simulink model "os_polyphase_synth_4096c"  (R2021a) (continued)
== system os_polyphase_synth_4096c/pfb_fir/pol1_in1_tap53 == (continued)
      | BCIN*ACIN+PCIN>>17+C
      | BCIN*ACIN+PCIN>>17+C+CARRYIN
      | BCIN*ACIN+PCIN>>17+CARRYIN
      | BCIN*ACIN+PCIN>>17+P
      | BCIN*ACIN+PCIN>>17+P+CARRYIN
      | BCIN*ACIN-C
      | BCIN*ACIN-P
      | BCIN*ACIN-P>>17
      | BCIN*ACIN-PCIN
      | BCIN*ACIN-PCIN>>17
      | BCIN*D
      | BCIN*D+C
      | BCIN*D+C+C
      | BCIN*D+C+C+CARRYIN
      | BCIN*D+C+CARRYIN
      | BCIN*D+C+P
      | BCIN*D+C+P+CARRYIN
      | BCIN*D+C+P>>17
      | BCIN*D+C+P>>17+CARRYIN
      | BCIN*D+C+PCIN
      | BCIN*D+C+PCIN+CARRYIN
      | BCIN*D+C+PCIN>>17
      | BCIN*D+C+PCIN>>17+CARRYIN
      | BCIN*D+C-C
      | BCIN*D+C-P
      | BCIN*D+C-P>>17
      | BCIN*D+C-PCIN
      | BCIN*D+C-PCIN>>17
      | BCIN*D+CARRYIN
      | BCIN*D+P
      | BCIN*D+P+C
      | BCIN*D+P+C+CARRYIN
      | BCIN*D+P+CARRYIN
      | BCIN*D+P+P
      | BCIN*D+P+P+CARRYIN
      | BCIN*D+P+P>>17
      | BCIN*D+P+P>>17+CARRYIN
      | BCIN*D+P+PCIN
      | BCIN*D+P+PCIN+CARRYIN
      | BCIN*D+P+PCIN>>17
      | BCIN*D+P+PCIN>>17+CARRYIN
      | BCIN*D+P-C
      | BCIN*D+P-P
      | BCIN*D+P-P>>17
      | BCIN*D+P-PCIN
      | BCIN*D+P-PCIN>>17
      | BCIN*D+P>>17
      | BCIN*D+P>>17+C
      | BCIN*D+P>>17+C+CARRYIN
      | BCIN*D+P>>17+CARRYIN
      | BCIN*D+P>>17+P
      | BCIN*D+P>>17+P+CARRYIN
      | BCIN*D+PCIN
      | BCIN*D+PCIN+C
      | BCIN*D+PCIN+C+CARRYIN
      | BCIN*D+PCIN+CARRYIN
      | BCIN*D+PCIN+P
      | BCIN*D+PCIN+P+CARRYIN
      | BCIN*D+PCIN>>17
      | BCIN*D+PCIN>>17+C
      | BCIN*D+PCIN>>17+C+CARRYIN
      | BCIN*D+PCIN>>17+CARRYIN
      | BCIN*D+PCIN>>17+P
      | BCIN*D+PCIN>>17+P+CARRYIN
      | BCIN*D-C
      | BCIN*D-P
      | BCIN*D-P>>17
      | BCIN*D-PCIN
      | BCIN*D-PCIN>>17
      | C
      | C+(A+D)
      | C+(A+D)*(A+D)
      | C+(A+D)*(A+D)+C
      | C+(A+D)*(A+D)+C+CARRYIN
      | C+(A+D)*(A+D)+CARRYIN
      | C+(A+D)*(A+D)+P
      | C+(A+D)*(A+D)+P+CARRYIN
      | C+(A+D)*(A+D)+P>>17
      | C+(A+D)*(A+D)+P>>17+CARRYIN
      | C+(A+D)*(A+D)+PCIN
      | C+(A+D)*(A+D)+PCIN+CARRYIN
      | C+(A+D)*(A+D)+PCIN>>17
      | C+(A+D)*(A+D)+PCIN>>17+CARRYIN
      | C+(A+D)*(A+D)-C
      | C+(A+D)*(A+D)-P
      | C+(A+D)*(A+D)-P>>17
      | C+(A+D)*(A+D)-PCIN
      | C+(A+D)*(A+D)-PCIN>>17
      | C+(A+D)*B
      | C+(A+D)*B+C
      | C+(A+D)*B+C+CARRYIN
      | C+(A+D)*B+CARRYIN
      | C+(A+D)*B+P
      | C+(A+D)*B+P+CARRYIN
      | C+(A+D)*B+P>>17
      | C+(A+D)*B+P>>17+CARRYIN
      | C+(A+D)*B+PCIN
      | C+(A+D)*B+PCIN+CARRYIN
      | C+(A+D)*B+PCIN>>17
      | C+(A+D)*B+PCIN>>17+CARRYIN
      | C+(A+D)*B-C
      | C+(A+D)*B-P
      | C+(A+D)*B-P>>17
      | C+(A+D)*B-PCIN
      | C+(A+D)*B-PCIN>>17
      | C+(A+D)*BCIN
      | C+(A+D)*BCIN+C
      | C+(A+D)*BCIN+C+CARRYIN
      | C+(A+D)*BCIN+CARRYIN
      | C+(A+D)*BCIN+P
      | C+(A+D)*BCIN+P+CARRYIN
      | C+(A+D)*BCIN+P>>17
      | C+(A+D)*BCIN+P>>17+CARRYIN
      | C+(A+D)*BCIN+PCIN
      | C+(A+D)*BCIN+PCIN+CARRYIN
      | C+(A+D)*BCIN+PCIN>>17
      | C+(A+D)*BCIN+PCIN>>17+CARRYIN
      | C+(A+D)*BCIN-C
      | C+(A+D)*BCIN-P
      | C+(A+D)*BCIN-P>>17
      | C+(A+D)*BCIN-PCIN
      | C+(A+D)*BCIN-PCIN>>17
      | C+(A+D)+C
      | C+(A+D)+C+CARRYIN
      | C+(A+D)+CARRYIN
      | C+(A+D)+P
      | C+(A+D)+P+CARRYIN
      | C+(A+D)+P>>17
      | C+(A+D)+P>>17+CARRYIN
      | C+(A+D)+PCIN
      | C+(A+D)+PCIN+CARRYIN
      | C+(A+D)+PCIN>>17
      | C+(A+D)+PCIN>>17+CARRYIN
      | C+(A+D)-C
      | C+(A+D)-P
      | C+(A+D)-P>>17
      | C+(A+D)-PCIN
      | C+(A+D)-PCIN>>17
      | C+(ACIN+D)
      | C+(ACIN+D)*(ACIN+D)
      | C+(ACIN+D)*(ACIN+D)+C
      | C+(ACIN+D)*(ACIN+D)+C+CARRYIN
      | C+(ACIN+D)*(ACIN+D)+CARRYIN
      | C+(ACIN+D)*(ACIN+D)+P
      | C+(ACIN+D)*(ACIN+D)+P+CARRYIN
      | C+(ACIN+D)*(ACIN+D)+P>>17
      | C+(ACIN+D)*(ACIN+D)+P>>17+CARRYIN
      | C+(ACIN+D)*(ACIN+D)+PCIN
      | C+(ACIN+D)*(ACIN+D)+PCIN+CARRYIN
      | C+(ACIN+D)*(ACIN+D)+PCIN>>17
      | C+(ACIN+D)*(ACIN+D)+PCIN>>17+CARRYIN
      | C+(ACIN+D)*(ACIN+D)-C
      | C+(ACIN+D)*(ACIN+D)-P
      | C+(ACIN+D)*(ACIN+D)-P>>17
      | C+(ACIN+D)*(ACIN+D)-PCIN
      | C+(ACIN+D)*(ACIN+D)-PCIN>>17
      | C+(ACIN+D)*B
      | C+(ACIN+D)*B+C
      | C+(ACIN+D)*B+C+CARRYIN
      | C+(ACIN+D)*B+CARRYIN
      | C+(ACIN+D)*B+P
      | C+(ACIN+D)*B+P+CARRYIN
      | C+(ACIN+D)*B+P>>17
      | C+(ACIN+D)*B+P>>17+CARRYIN
      | C+(ACIN+D)*B+PCIN
      | C+(ACIN+D)*B+PCIN+CARRYIN
      | C+(ACIN+D)*B+PCIN>>17
      | C+(ACIN+D)*B+PCIN>>17+CARRYIN
      | C+(ACIN+D)*B-C
      | C+(ACIN+D)*B-P
      | C+(ACIN+D)*B-P>>17
      | C+(ACIN+D)*B-PCIN
      | C+(ACIN+D)*B-PCIN>>17
      | C+(ACIN+D)*BCIN
      | C+(ACIN+D)*BCIN+C
      | C+(ACIN+D)*BCIN+C+CARRYIN
      | C+(ACIN+D)*BCIN+CARRYIN
      | C+(ACIN+D)*BCIN+P
      | C+(ACIN+D)*BCIN+P+CARRYIN
      | C+(ACIN+D)*BCIN+P>>17
      | C+(ACIN+D)*BCIN+P>>17+CARRYIN
      | C+(ACIN+D)*BCIN+PCIN
      | C+(ACIN+D)*BCIN+PCIN+CARRYIN
      | C+(ACIN+D)*BCIN+PCIN>>17
      | C+(ACIN+D)*BCIN+PCIN>>17+CARRYIN
      | C+(ACIN+D)*BCIN-C
      | C+(ACIN+D)*BCIN-P
      | C+(ACIN+D)*BCIN-P>>17
      | C+(ACIN+D)*BCIN-PCIN
      | C+(ACIN+D)*BCIN-PCIN>>17
      | C+(ACIN+D)+C
      | C+(ACIN+D)+C+CARRYIN
      | C+(ACIN+D)+CARRYIN
      | C+(ACIN+D)+P
      | C+(ACIN+D)+P+CARRYIN
      | C+(ACIN+D)+P>>17
      | C+(ACIN+D)+P>>17+CARRYIN
      | C+(ACIN+D)+PCIN
      | C+(ACIN+D)+PCIN+CARRYIN
      | C+(ACIN+D)+PCIN>>17
      | C+(ACIN+D)+PCIN>>17+CARRYIN
      | C+(ACIN+D)-C
      | C+(ACIN+D)-P
      | C+(ACIN+D)-P>>17
      | C+(ACIN+D)-PCIN
      | C+(ACIN+D)-PCIN>>17
      | C+(D+A)
      | C+(D+A)*(D+A)
      | C+(D+A)*(D+A)+C
      | C+(D+A)*(D+A)+C+CARRYIN
      | C+(D+A)*(D+A)+CARRYIN
      | C+(D+A)*(D+A)+P
      | C+(D+A)*(D+A)+P+CARRYIN
      | C+(D+A)*(D+A)+P>>17
      | C+(D+A)*(D+A)+P>>17+CARRYIN
      | C+(D+A)*(D+A)+PCIN
      | C+(D+A)*(D+A)+PCIN+CARRYIN
      | C+(D+A)*(D+A)+PCIN>>17
      | C+(D+A)*(D+A)+PCIN>>17+CARRYIN
      | C+(D+A)*(D+A)-C
      | C+(D+A)*(D+A)-P
      | C+(D+A)*(D+A)-P>>17
      | C+(D+A)*(D+A)-PCIN
      | C+(D+A)*(D+A)-PCIN>>17
      | C+(D+A)*B
      | C+(D+A)*B+C
      | C+(D+A)*B+C+CARRYIN
      | C+(D+A)*B+CARRYIN
      | C+(D+A)*B+P
      | C+(D+A)*B+P+CARRYIN
      | C+(D+A)*B+P>>17
      | C+(D+A)*B+P>>17+CARRYIN
      | C+(D+A)*B+PCIN
      | C+(D+A)*B+PCIN+CARRYIN
      | C+(D+A)*B+PCIN>>17
      | C+(D+A)*B+PCIN>>17+CARRYIN
      | C+(D+A)*B-C
      | C+(D+A)*B-P
      | C+(D+A)*B-P>>17
      | C+(D+A)*B-PCIN
      | C+(D+A)*B-PCIN>>17
      | C+(D+A)*BCIN
      | C+(D+A)*BCIN+C
      | C+(D+A)*BCIN+C+CARRYIN
      | C+(D+A)*BCIN+CARRYIN
      | C+(D+A)*BCIN+P
      | C+(D+A)*BCIN+P+CARRYIN
      | C+(D+A)*BCIN+P>>17
      | C+(D+A)*BCIN+P>>17+CARRYIN
      | C+(D+A)*BCIN+PCIN
      | C+(D+A)*BCIN+PCIN+CARRYIN
      | C+(D+A)*BCIN+PCIN>>17
      | C+(D+A)*BCIN+PCIN>>17+CARRYIN
      | C+(D+A)*BCIN-C
      | C+(D+A)*BCIN-P
      | C+(D+A)*BCIN-P>>17
      | C+(D+A)*BCIN-PCIN
      | C+(D+A)*BCIN-PCIN>>17
      | C+(D+A)+C
      | C+(D+A)+C+CARRYIN
      | C+(D+A)+CARRYIN
      | C+(D+A)+P
      | C+(D+A)+P+CARRYIN
      | C+(D+A)+P>>17
      | C+(D+A)+P>>17+CARRYIN
      | C+(D+A)+PCIN
      | C+(D+A)+PCIN+CARRYIN
      | C+(D+A)+PCIN>>17
      | C+(D+A)+PCIN>>17+CARRYIN
      | C+(D+A)-C
      | C+(D+A)-P
      | C+(D+A)-P>>17
      | C+(D+A)-PCIN
      | C+(D+A)-PCIN>>17
      | C+(D+ACIN)
      | C+(D+ACIN)*(D+ACIN)
      | C+(D+ACIN)*(D+ACIN)+C
      | C+(D+ACIN)*(D+ACIN)+C+CARRYIN
      | C+(D+ACIN)*(D+ACIN)+CARRYIN
      | C+(D+ACIN)*(D+ACIN)+P
      | C+(D+ACIN)*(D+ACIN)+P+CARRYIN
      | C+(D+ACIN)*(D+ACIN)+P>>17
      | C+(D+ACIN)*(D+ACIN)+P>>17+CARRYIN
      | C+(D+ACIN)*(D+ACIN)+PCIN
      | C+(D+ACIN)*(D+ACIN)+PCIN+CARRYIN
      | C+(D+ACIN)*(D+ACIN)+PCIN>>17
      | C+(D+ACIN)*(D+ACIN)+PCIN>>17+CARRYIN
      | C+(D+ACIN)*(D+ACIN)-C
      | C+(D+ACIN)*(D+ACIN)-P
      | C+(D+ACIN)*(D+ACIN)-P>>17
      | C+(D+ACIN)*(D+ACIN)-PCIN
      | C+(D+ACIN)*(D+ACIN)-PCIN>>17
      | C+(D+ACIN)*B
      | C+(D+ACIN)*B+C
      | C+(D+ACIN)*B+C+CARRYIN
      | C+(D+ACIN)*B+CARRYIN
      | C+(D+ACIN)*B+P
      | C+(D+ACIN)*B+P+CARRYIN
      | C+(D+ACIN)*B+P>>17
      | C+(D+ACIN)*B+P>>17+CARRYIN
      | C+(D+ACIN)*B+PCIN
      | C+(D+ACIN)*B+PCIN+CARRYIN
      | C+(D+ACIN)*B+PCIN>>17
      | C+(D+ACIN)*B+PCIN>>17+CARRYIN
      | C+(D+ACIN)*B-C
      | C+(D+ACIN)*B-P
      | C+(D+ACIN)*B-P>>17
      | C+(D+ACIN)*B-PCIN
      | C+(D+ACIN)*B-PCIN>>17
      | C+(D+ACIN)*BCIN
      | C+(D+ACIN)*BCIN+C
      | C+(D+ACIN)*BCIN+C+CARRYIN
      | C+(D+ACIN)*BCIN+CARRYIN
      | C+(D+ACIN)*BCIN+P
      | C+(D+ACIN)*BCIN+P+CARRYIN
      | C+(D+ACIN)*BCIN+P>>17
      | C+(D+ACIN)*BCIN+P>>17+CARRYIN
      | C+(D+ACIN)*BCIN+PCIN
      | C+(D+ACIN)*BCIN+PCIN+CARRYIN
      | C+(D+ACIN)*BCIN+PCIN>>17
      | C+(D+ACIN)*BCIN+PCIN>>17+CARRYIN
      | C+(D+ACIN)*BCIN-C
      | C+(D+ACIN)*BCIN-P
      | C+(D+ACIN)*BCIN-P>>17
      | C+(D+ACIN)*BCIN-PCIN
      | C+(D+ACIN)*BCIN-PCIN>>17
      | C+(D+ACIN)+C
      | C+(D+ACIN)+C+CARRYIN
      | C+(D+ACIN)+CARRYIN
      | C+(D+ACIN)+P
      | C+(D+ACIN)+P+CARRYIN
      | C+(D+ACIN)+P>>17
      | C+(D+ACIN)+P>>17+CARRYIN
      | C+(D+ACIN)+PCIN
      | C+(D+ACIN)+PCIN+CARRYIN
      | C+(D+ACIN)+PCIN>>17
      | C+(D+ACIN)+PCIN>>17+CARRYIN
      | C+(D+ACIN)-C
      | C+(D+ACIN)-P
      | C+(D+ACIN)-P>>17
      | C+(D+ACIN)-PCIN
      | C+(D+ACIN)-PCIN>>17
      | C+(D-A)
      | C+(D-A)*(D-A)
      | C+(D-A)*(D-A)+C
      | C+(D-A)*(D-A)+C+CARRYIN
      | C+(D-A)*(D-A)+CARRYIN
      | C+(D-A)*(D-A)+P
      | C+(D-A)*(D-A)+P+CARRYIN
      | C+(D-A)*(D-A)+P>>17
      | C+(D-A)*(D-A)+P>>17+CARRYIN
      | C+(D-A)*(D-A)+PCIN
      | C+(D-A)*(D-A)+PCIN+CARRYIN
      | C+(D-A)*(D-A)+PCIN>>17
      | C+(D-A)*(D-A)+PCIN>>17+CARRYIN
      | C+(D-A)*(D-A)-C
      | C+(D-A)*(D-A)-P
      | C+(D-A)*(D-A)-P>>17
      | C+(D-A)*(D-A)-PCIN
      | C+(D-A)*(D-A)-PCIN>>17
      | C+(D-A)*B
      | C+(D-A)*B+C
      | C+(D-A)*B+C+CARRYIN
      | C+(D-A)*B+CARRYIN
      | C+(D-A)*B+P
      | C+(D-A)*B+P+CARRYIN
      | C+(D-A)*B+P>>17
      | C+(D-A)*B+P>>17+CARRYIN
      | C+(D-A)*B+PCIN
      | C+(D-A)*B+PCIN+CARRYIN
      | C+(D-A)*B+PCIN>>17
      | C+(D-A)*B+PCIN>>17+CARRYIN
      | C+(D-A)*B-C
      | C+(D-A)*B-P
      | C+(D-A)*B-P>>17
      | C+(D-A)*B-PCIN
      | C+(D-A)*B-PCIN>>17
      | C+(D-A)*BCIN
      | C+(D-A)*BCIN+C
      | C+(D-A)*BCIN+C+CARRYIN
      | C+(D-A)*BCIN+CARRYIN
      | C+(D-A)*BCIN+P
      | C+(D-A)*BCIN+P+CARRYIN
      | C+(D-A)*BCIN+P>>17
      | C+(D-A)*BCIN+P>>17+CARRYIN
      | C+(D-A)*BCIN+PCIN
      | C+(D-A)*BCIN+PCIN+CARRYIN
      | C+(D-A)*BCIN+PCIN>>17
      | C+(D-A)*BCIN+PCIN>>17+CARRYIN
      | C+(D-A)*BCIN-C
      | C+(D-A)*BCIN-P
      | C+(D-A)*BCIN-P>>17
      | C+(D-A)*BCIN-PCIN
      | C+(D-A)*BCIN-PCIN>>17
      | C+(D-A)+C
      | C+(D-A)+C+CARRYIN
      | C+(D-A)+CARRYIN
      | C+(D-A)+P
      | C+(D-A)+P+CARRYIN
      | C+(D-A)+P>>17
      | C+(D-A)+P>>17+CARRYIN
      | C+(D-A)+PCIN
      | C+(D-A)+PCIN+CARRYIN
      | C+(D-A)+PCIN>>17
      | C+(D-A)+PCIN>>17+CARRYIN
      | C+(D-A)-C
      | C+(D-A)-P
      | C+(D-A)-P>>17
      | C+(D-A)-PCIN
      | C+(D-A)-PCIN>>17
      | C+(D-ACIN)
      | C+(D-ACIN)*(D-ACIN)
      | C+(D-ACIN)*(D-ACIN)+C
      | C+(D-ACIN)*(D-ACIN)+C+CARRYIN
      | C+(D-ACIN)*(D-ACIN)+CARRYIN
      | C+(D-ACIN)*(D-ACIN)+P
      | C+(D-ACIN)*(D-ACIN)+P+CARRYIN
      | C+(D-ACIN)*(D-ACIN)+P>>17
      | C+(D-ACIN)*(D-ACIN)+P>>17+CARRYIN
      | C+(D-ACIN)*(D-ACIN)+PCIN
      | C+(D-ACIN)*(D-ACIN)+PCIN+CARRYIN
      | C+(D-ACIN)*(D-ACIN)+PCIN>>17
      | C+(D-ACIN)*(D-ACIN)+PCIN>>17+CARRYIN
      | C+(D-ACIN)*(D-ACIN)-C
      | C+(D-ACIN)*(D-ACIN)-P
      | C+(D-ACIN)*(D-ACIN)-P>>17
      | C+(D-ACIN)*(D-ACIN)-PCIN
      | C+(D-ACIN)*(D-ACIN)-PCIN>>17
      | C+(D-ACIN)*B
      | C+(D-ACIN)*B+C
      | C+(D-ACIN)*B+C+CARRYIN
      | C+(D-ACIN)*B+CARRYIN
      | C+(D-ACIN)*B+P
      | C+(D-ACIN)*B+P+CARRYIN
      | C+(D-ACIN)*B+P>>17
      | C+(D-ACIN)*B+P>>17+CARRYIN
      | C+(D-ACIN)*B+PCIN
      | C+(D-ACIN)*B+PCIN+CARRYIN
      | C+(D-ACIN)*B+PCIN>>17
      | C+(D-ACIN)*B+PCIN>>17+CARRYIN
      | C+(D-ACIN)*B-C
      | C+(D-ACIN)*B-P
      | C+(D-ACIN)*B-P>>17
      | C+(D-ACIN)*B-PCIN
      | C+(D-ACIN)*B-PCIN>>17
      | C+(D-ACIN)*BCIN
      | C+(D-ACIN)*BCIN+C
      | C+(D-ACIN)*BCIN+C+CARRYIN
      | C+(D-ACIN)*BCIN+CARRYIN
      | C+(D-ACIN)*BCIN+P
      | C+(D-ACIN)*BCIN+P+CARRYIN
      | C+(D-ACIN)*BCIN+P>>17
      | C+(D-ACIN)*BCIN+P>>17+CARRYIN
      | C+(D-ACIN)*BCIN+PCIN
      | C+(D-ACIN)*BCIN+PCIN+CARRYIN
      | C+(D-ACIN)*BCIN+PCIN>>17
      | C+(D-ACIN)*BCIN+PCIN>>17+CARRYIN
      | C+(D-ACIN)*BCIN-C
      | C+(D-ACIN)*BCIN-P
      | C+(D-ACIN)*BCIN-P>>17
      | C+(D-ACIN)*BCIN-PCIN
      | C+(D-ACIN)*BCIN-PCIN>>17
      | C+(D-ACIN)+C
      | C+(D-ACIN)+C+CARRYIN
      | C+(D-ACIN)+CARRYIN
      | C+(D-ACIN)+P
      | C+(D-ACIN)+P+CARRYIN
      | C+(D-ACIN)+P>>17
      | C+(D-ACIN)+P>>17+CARRYIN
      | C+(D-ACIN)+PCIN
      | C+(D-ACIN)+PCIN+CARRYIN
      | C+(D-ACIN)+PCIN>>17
      | C+(D-ACIN)+PCIN>>17+CARRYIN
      | C+(D-ACIN)-C
      | C+(D-ACIN)-P
      | C+(D-ACIN)-P>>17
      | C+(D-ACIN)-PCIN
      | C+(D-ACIN)-PCIN>>17
      | C+A
      | C+A*A
      | C+A*A+C
      | C+A*A+C+CARRYIN
      | C+A*A+CARRYIN
      | C+A*A+P
      | C+A*A+P+CARRYIN
      | C+A*A+P>>17
      | C+A*A+P>>17+CARRYIN
      | C+A*A+PCIN
      | C+A*A+PCIN+CARRYIN
      | C+A*A+PCIN>>17
      | C+A*A+PCIN>>17+CARRYIN
      | C+A*A-C
      | C+A*A-P
      | C+A*A-P>>17
      | C+A*A-PCIN
      | C+A*A-PCIN>>17
      | C+A*B
      | C+A*B+C
      | C+A*B+C+CARRYIN
      | C+A*B+CARRYIN
      | C+A*B+P
      | C+A*B+P+CARRYIN
      | C+A*B+P>>17
      | C+A*B+P>>17+CARRYIN
      | C+A*B+PCIN
      | C+A*B+PCIN+CARRYIN
      | C+A*B+PCIN>>17
      | C+A*B+PCIN>>17+CARRYIN
      | C+A*B-C
      | C+A*B-P
      | C+A*B-P>>17
      | C+A*B-PCIN
      | C+A*B-PCIN>>17
      | C+A*BCIN
      | C+A*BCIN+C
      | C+A*BCIN+C+CARRYIN
      | C+A*BCIN+CARRYIN
      | C+A*BCIN+P
      | C+A*BCIN+P+CARRYIN
      | C+A*BCIN+P>>17
      | C+A*BCIN+P>>17+CARRYIN
      | C+A*BCIN+PCIN
      | C+A*BCIN+PCIN+CARRYIN
      | C+A*BCIN+PCIN>>17
      | C+A*BCIN+PCIN>>17+CARRYIN
      | C+A*BCIN-C
      | C+A*BCIN-P
      | C+A*BCIN-P>>17
      | C+A*BCIN-PCIN
      | C+A*BCIN-PCIN>>17
      | C+A+C
      | C+A+C+CARRYIN
      | C+A+CARRYIN
      | C+A+P
      | C+A+P+CARRYIN
      | C+A+P>>17
      | C+A+P>>17+CARRYIN
      | C+A+PCIN
      | C+A+PCIN+CARRYIN
      | C+A+PCIN>>17
      | C+A+PCIN>>17+CARRYIN
      | C+A-C
      | C+A-P
      | C+A-P>>17
      | C+A-PCIN
      | C+A-PCIN>>17
      | C+ACIN
      | C+ACIN*ACIN
      | C+ACIN*ACIN+C
      | C+ACIN*ACIN+C+CARRYIN
      | C+ACIN*ACIN+CARRYIN
      | C+ACIN*ACIN+P
      | C+ACIN*ACIN+P+CARRYIN
      | C+ACIN*ACIN+P>>17
      | C+ACIN*ACIN+P>>17+CARRYIN
      | C+ACIN*ACIN+PCIN
      | C+ACIN*ACIN+PCIN+CARRYIN
      | C+ACIN*ACIN+PCIN>>17
      | C+ACIN*ACIN+PCIN>>17+CARRYIN
      | C+ACIN*ACIN-C
      | C+ACIN*ACIN-P
      | C+ACIN*ACIN-P>>17
      | C+ACIN*ACIN-PCIN
      | C+ACIN*ACIN-PCIN>>17
      | C+ACIN*B
      | C+ACIN*B+C
      | C+ACIN*B+C+CARRYIN
      | C+ACIN*B+CARRYIN
      | C+ACIN*B+P
      | C+ACIN*B+P+CARRYIN
      | C+ACIN*B+P>>17
      | C+ACIN*B+P>>17+CARRYIN
      | C+ACIN*B+PCIN
      | C+ACIN*B+PCIN+CARRYIN
      | C+ACIN*B+PCIN>>17
      | C+ACIN*B+PCIN>>17+CARRYIN
      | C+ACIN*B-C
      | C+ACIN*B-P
      | C+ACIN*B-P>>17
      | C+ACIN*B-PCIN
      | C+ACIN*B-PCIN>>17
      | C+ACIN*BCIN
      | C+ACIN*BCIN+C
      | C+ACIN*BCIN+C+CARRYIN
      | C+ACIN*BCIN+CARRYIN
      | C+ACIN*BCIN+P
      | C+ACIN*BCIN+P+CARRYIN
      | C+ACIN*BCIN+P>>17
      | C+ACIN*BCIN+P>>17+CARRYIN
      | C+ACIN*BCIN+PCIN
      | C+ACIN*BCIN+PCIN+CARRYIN
      | C+ACIN*BCIN+PCIN>>17
      | C+ACIN*BCIN+PCIN>>17+CARRYIN
      | C+ACIN*BCIN-C
      | C+ACIN*BCIN-P
      | C+ACIN*BCIN-P>>17
      | C+ACIN*BCIN-PCIN
      | C+ACIN*BCIN-PCIN>>17
      | C+ACIN+C
      | C+ACIN+C+CARRYIN
      | C+ACIN+CARRYIN
      | C+ACIN+P
      | C+ACIN+P+CARRYIN
      | C+ACIN+P>>17
      | C+ACIN+P>>17+CARRYIN
      | C+ACIN+PCIN
      | C+ACIN+PCIN+CARRYIN
      | C+ACIN+PCIN>>17
      | C+ACIN+PCIN>>17+CARRYIN
      | C+ACIN-C
      | C+ACIN-P
      | C+ACIN-P>>17
      | C+ACIN-PCIN
      | C+ACIN-PCIN>>17
      | C+B*(A+D)
      | C+B*(A+D)+C
      | C+B*(A+D)+C+CARRYIN
      | C+B*(A+D)+CARRYIN
      | C+B*(A+D)+P
      | C+B*(A+D)+P+CARRYIN
      | C+B*(A+D)+P>>17
      | C+B*(A+D)+P>>17+CARRYIN
      | C+B*(A+D)+PCIN
      | C+B*(A+D)+PCIN+CARRYIN
      | C+B*(A+D)+PCIN>>17
      | C+B*(A+D)+PCIN>>17+CARRYIN
      | C+B*(A+D)-C
      | C+B*(A+D)-P
      | C+B*(A+D)-P>>17
      | C+B*(A+D)-PCIN
      | C+B*(A+D)-PCIN>>17
      | C+B*(ACIN+D)
      | C+B*(ACIN+D)+C
      | C+B*(ACIN+D)+C+CARRYIN
      | C+B*(ACIN+D)+CARRYIN
      | C+B*(ACIN+D)+P
      | C+B*(ACIN+D)+P+CARRYIN
      | C+B*(ACIN+D)+P>>17
      | C+B*(ACIN+D)+P>>17+CARRYIN
      | C+B*(ACIN+D)+PCIN
      | C+B*(ACIN+D)+PCIN+CARRYIN
      | C+B*(ACIN+D)+PCIN>>17
      | C+B*(ACIN+D)+PCIN>>17+CARRYIN
      | C+B*(ACIN+D)-C
      | C+B*(ACIN+D)-P
      | C+B*(ACIN+D)-P>>17
      | C+B*(ACIN+D)-PCIN
      | C+B*(ACIN+D)-PCIN>>17
      | C+B*(D+A)
      | C+B*(D+A)+C
      | C+B*(D+A)+C+CARRYIN
      | C+B*(D+A)+CARRYIN
      | C+B*(D+A)+P
      | C+B*(D+A)+P+CARRYIN
      | C+B*(D+A)+P>>17
      | C+B*(D+A)+P>>17+CARRYIN
      | C+B*(D+A)+PCIN
      | C+B*(D+A)+PCIN+CARRYIN
      | C+B*(D+A)+PCIN>>17
      | C+B*(D+A)+PCIN>>17+CARRYIN
      | C+B*(D+A)-C
      | C+B*(D+A)-P
      | C+B*(D+A)-P>>17
      | C+B*(D+A)-PCIN
      | C+B*(D+A)-PCIN>>17
      | C+B*(D+ACIN)
      | C+B*(D+ACIN)+C
      | C+B*(D+ACIN)+C+CARRYIN
      | C+B*(D+ACIN)+CARRYIN
      | C+B*(D+ACIN)+P
      | C+B*(D+ACIN)+P+CARRYIN
      | C+B*(D+ACIN)+P>>17
      | C+B*(D+ACIN)+P>>17+CARRYIN
      | C+B*(D+ACIN)+PCIN
      | C+B*(D+ACIN)+PCIN+CARRYIN
      | C+B*(D+ACIN)+PCIN>>17
      | C+B*(D+ACIN)+PCIN>>17+CARRYIN
      | C+B*(D+ACIN)-C
      | C+B*(D+ACIN)-P
      | C+B*(D+ACIN)-P>>17
      | C+B*(D+ACIN)-PCIN
      | C+B*(D+ACIN)-PCIN>>17
      | C+B*(D-A)
      | C+B*(D-A)+C
      | C+B*(D-A)+C+CARRYIN
      | C+B*(D-A)+CARRYIN
      | C+B*(D-A)+P
      | C+B*(D-A)+P+CARRYIN
      | C+B*(D-A)+P>>17
      | C+B*(D-A)+P>>17+CARRYIN
      | C+B*(D-A)+PCIN
      | C+B*(D-A)+PCIN+CARRYIN
      | C+B*(D-A)+PCIN>>17
      | C+B*(D-A)+PCIN>>17+CARRYIN
      | C+B*(D-A)-C
      | C+B*(D-A)-P
      | C+B*(D-A)-P>>17
      | C+B*(D-A)-PCIN
      | C+B*(D-A)-PCIN>>17
      | C+B*(D-ACIN)
      | C+B*(D-ACIN)+C
      | C+B*(D-ACIN)+C+CARRYIN
      | C+B*(D-ACIN)+CARRYIN
      | C+B*(D-ACIN)+P
      | C+B*(D-ACIN)+P+CARRYIN
      | C+B*(D-ACIN)+P>>17
      | C+B*(D-ACIN)+P>>17+CARRYIN
      | C+B*(D-ACIN)+PCIN
      | C+B*(D-ACIN)+PCIN+CARRYIN
      | C+B*(D-ACIN)+PCIN>>17
      | C+B*(D-ACIN)+PCIN>>17+CARRYIN
      | C+B*(D-ACIN)-C
      | C+B*(D-ACIN)-P
      | C+B*(D-ACIN)-P>>17
      | C+B*(D-ACIN)-PCIN
      | C+B*(D-ACIN)-PCIN>>17
      | C+B*A
      | C+B*A+C
      | C+B*A+C+CARRYIN
      | C+B*A+CARRYIN
      | C+B*A+P
      | C+B*A+P+CARRYIN
      | C+B*A+P>>17
      | C+B*A+P>>17+CARRYIN
      | C+B*A+PCIN
      | C+B*A+PCIN+CARRYIN
      | C+B*A+PCIN>>17
      | C+B*A+PCIN>>17+CARRYIN
      | C+B*A-C
      | C+B*A-P
      | C+B*A-P>>17
      | C+B*A-PCIN
      | C+B*A-PCIN>>17
      | C+B*ACIN
      | C+B*ACIN+C
      | C+B*ACIN+C+CARRYIN
      | C+B*ACIN+CARRYIN
      | C+B*ACIN+P
      | C+B*ACIN+P+CARRYIN
      | C+B*ACIN+P>>17
      | C+B*ACIN+P>>17+CARRYIN
      | C+B*ACIN+PCIN
      | C+B*ACIN+PCIN+CARRYIN
      | C+B*ACIN+PCIN>>17
      | C+B*ACIN+PCIN>>17+CARRYIN
      | C+B*ACIN-C
      | C+B*ACIN-P
      | C+B*ACIN-P>>17
      | C+B*ACIN-PCIN
      | C+B*ACIN-PCIN>>17
      | C+B*D
      | C+B*D+C
      | C+B*D+C+CARRYIN
      | C+B*D+CARRYIN
      | C+B*D+P
      | C+B*D+P+CARRYIN
      | C+B*D+P>>17
      | C+B*D+P>>17+CARRYIN
      | C+B*D+PCIN
      | C+B*D+PCIN+CARRYIN
      | C+B*D+PCIN>>17
      | C+B*D+PCIN>>17+CARRYIN
      | C+B*D-C
      | C+B*D-P
      | C+B*D-P>>17
      | C+B*D-PCIN
      | C+B*D-PCIN>>17
      | C+BCIN*(A+D)
      | C+BCIN*(A+D)+C
      | C+BCIN*(A+D)+C+CARRYIN
      | C+BCIN*(A+D)+CARRYIN
      | C+BCIN*(A+D)+P
      | C+BCIN*(A+D)+P+CARRYIN
      | C+BCIN*(A+D)+P>>17
      | C+BCIN*(A+D)+P>>17+CARRYIN
      | C+BCIN*(A+D)+PCIN
      | C+BCIN*(A+D)+PCIN+CARRYIN
      | C+BCIN*(A+D)+PCIN>>17
      | C+BCIN*(A+D)+PCIN>>17+CARRYIN
      | C+BCIN*(A+D)-C
      | C+BCIN*(A+D)-P
      | C+BCIN*(A+D)-P>>17
      | C+BCIN*(A+D)-PCIN
      | C+BCIN*(A+D)-PCIN>>17
      | C+BCIN*(ACIN+D)
      | C+BCIN*(ACIN+D)+C
      | C+BCIN*(ACIN+D)+C+CARRYIN
      | C+BCIN*(ACIN+D)+CARRYIN
      | C+BCIN*(ACIN+D)+P
      | C+BCIN*(ACIN+D)+P+CARRYIN
      | C+BCIN*(ACIN+D)+P>>17
      | C+BCIN*(ACIN+D)+P>>17+CARRYIN
      | C+BCIN*(ACIN+D)+PCIN
      | C+BCIN*(ACIN+D)+PCIN+CARRYIN
      | C+BCIN*(ACIN+D)+PCIN>>17
      | C+BCIN*(ACIN+D)+PCIN>>17+CARRYIN
      | C+BCIN*(ACIN+D)-C
      | C+BCIN*(ACIN+D)-P
      | C+BCIN*(ACIN+D)-P>>17
      | C+BCIN*(ACIN+D)-PCIN
      | C+BCIN*(ACIN+D)-PCIN>>17
      | C+BCIN*(D+A)
      | C+BCIN*(D+A)+C
      | C+BCIN*(D+A)+C+CARRYIN
      | C+BCIN*(D+A)+CARRYIN
      | C+BCIN*(D+A)+P
      | C+BCIN*(D+A)+P+CARRYIN
      | C+BCIN*(D+A)+P>>17
      | C+BCIN*(D+A)+P>>17+CARRYIN
      | C+BCIN*(D+A)+PCIN
      | C+BCIN*(D+A)+PCIN+CARRYIN
      | C+BCIN*(D+A)+PCIN>>17
      | C+BCIN*(D+A)+PCIN>>17+CARRYIN
      | C+BCIN*(D+A)-C
      | C+BCIN*(D+A)-P
      | C+BCIN*(D+A)-P>>17
      | C+BCIN*(D+A)-PCIN
      | C+BCIN*(D+A)-PCIN>>17
      | C+BCIN*(D+ACIN)
      | C+BCIN*(D+ACIN)+C
      | C+BCIN*(D+ACIN)+C+CARRYIN
      | C+BCIN*(D+ACIN)+CARRYIN
      | C+BCIN*(D+ACIN)+P
      | C+BCIN*(D+ACIN)+P+CARRYIN
      | C+BCIN*(D+ACIN)+P>>17
      | C+BCIN*(D+ACIN)+P>>17+CARRYIN
      | C+BCIN*(D+ACIN)+PCIN
      | C+BCIN*(D+ACIN)+PCIN+CARRYIN
      | C+BCIN*(D+ACIN)+PCIN>>17
      | C+BCIN*(D+ACIN)+PCIN>>17+CARRYIN
      | C+BCIN*(D+ACIN)-C
      | C+BCIN*(D+ACIN)-P
      | C+BCIN*(D+ACIN)-P>>17
      | C+BCIN*(D+ACIN)-PCIN
      | C+BCIN*(D+ACIN)-PCIN>>17
      | C+BCIN*(D-A)
      | C+BCIN*(D-A)+C
      | C+BCIN*(D-A)+C+CARRYIN
      | C+BCIN*(D-A)+CARRYIN
      | C+BCIN*(D-A)+P
      | C+BCIN*(D-A)+P+CARRYIN
      | C+BCIN*(D-A)+P>>17
      | C+BCIN*(D-A)+P>>17+CARRYIN
      | C+BCIN*(D-A)+PCIN
      | C+BCIN*(D-A)+PCIN+CARRYIN
      | C+BCIN*(D-A)+PCIN>>17
      | C+BCIN*(D-A)+PCIN>>17+CARRYIN
      | C+BCIN*(D-A)-C
      | C+BCIN*(D-A)-P
      | C+BCIN*(D-A)-P>>17
      | C+BCIN*(D-A)-PCIN
      | C+BCIN*(D-A)-PCIN>>17
      | C+BCIN*(D-ACIN)
      | C+BCIN*(D-ACIN)+C
      | C+BCIN*(D-ACIN)+C+CARRYIN
      | C+BCIN*(D-ACIN)+CARRYIN
      | C+BCIN*(D-ACIN)+P
      | C+BCIN*(D-ACIN)+P+CARRYIN
      | C+BCIN*(D-ACIN)+P>>17
      | C+BCIN*(D-ACIN)+P>>17+CARRYIN
      | C+BCIN*(D-ACIN)+PCIN
      | C+BCIN*(D-ACIN)+PCIN+CARRYIN
      | C+BCIN*(D-ACIN)+PCIN>>17
      | C+BCIN*(D-ACIN)+PCIN>>17+CARRYIN
      | C+BCIN*(D-ACIN)-C
      | C+BCIN*(D-ACIN)-P
      | C+BCIN*(D-ACIN)-P>>17
      | C+BCIN*(D-ACIN)-PCIN
      | C+BCIN*(D-ACIN)-PCIN>>17
      | C+BCIN*A
      | C+BCIN*A+C
      | C+BCIN*A+C+CARRYIN
      | C+BCIN*A+CARRYIN
      | C+BCIN*A+P
      | C+BCIN*A+P+CARRYIN
      | C+BCIN*A+P>>17
      | C+BCIN*A+P>>17+CARRYIN
      | C+BCIN*A+PCIN
      | C+BCIN*A+PCIN+CARRYIN
      | C+BCIN*A+PCIN>>17
      | C+BCIN*A+PCIN>>17+CARRYIN
      | C+BCIN*A-C
      | C+BCIN*A-P
      | C+BCIN*A-P>>17
      | C+BCIN*A-PCIN
      | C+BCIN*A-PCIN>>17
      | C+BCIN*ACIN
      | C+BCIN*ACIN+C
      | C+BCIN*ACIN+C+CARRYIN
      | C+BCIN*ACIN+CARRYIN
      | C+BCIN*ACIN+P
      | C+BCIN*ACIN+P+CARRYIN
      | C+BCIN*ACIN+P>>17
      | C+BCIN*ACIN+P>>17+CARRYIN
      | C+BCIN*ACIN+PCIN
      | C+BCIN*ACIN+PCIN+CARRYIN
      | C+BCIN*ACIN+PCIN>>17
      | C+BCIN*ACIN+PCIN>>17+CARRYIN
      | C+BCIN*ACIN-C
      | C+BCIN*ACIN-P
      | C+BCIN*ACIN-P>>17
      | C+BCIN*ACIN-PCIN
      | C+BCIN*ACIN-PCIN>>17
      | C+BCIN*D
      | C+BCIN*D+C
      | C+BCIN*D+C+CARRYIN
      | C+BCIN*D+CARRYIN
      | C+BCIN*D+P
      | C+BCIN*D+P+CARRYIN
      | C+BCIN*D+P>>17
      | C+BCIN*D+P>>17+CARRYIN
      | C+BCIN*D+PCIN
      | C+BCIN*D+PCIN+CARRYIN
      | C+BCIN*D+PCIN>>17
      | C+BCIN*D+PCIN>>17+CARRYIN
      | C+BCIN*D-C
      | C+BCIN*D-P
      | C+BCIN*D-P>>17
      | C+BCIN*D-PCIN
      | C+BCIN*D-PCIN>>17
      | C+C
      | C+C+(A+D)
      | C+C+(A+D)*(A+D)
      | C+C+(A+D)*(A+D)+CARRYIN
      | C+C+(A+D)*B
      | C+C+(A+D)*B+CARRYIN
      | C+C+(A+D)*BCIN
      | C+C+(A+D)*BCIN+CARRYIN
      | C+C+(A+D)+CARRYIN
      | C+C+(ACIN+D)
      | C+C+(ACIN+D)*(ACIN+D)
      | C+C+(ACIN+D)*(ACIN+D)+CARRYIN
      | C+C+(ACIN+D)*B
      | C+C+(ACIN+D)*B+CARRYIN
      | C+C+(ACIN+D)*BCIN
      | C+C+(ACIN+D)*BCIN+CARRYIN
      | C+C+(ACIN+D)+CARRYIN
      | C+C+(D+A)
      | C+C+(D+A)*(D+A)
      | C+C+(D+A)*(D+A)+CARRYIN
      | C+C+(D+A)*B
      | C+C+(D+A)*B+CARRYIN
      | C+C+(D+A)*BCIN
      | C+C+(D+A)*BCIN+CARRYIN
      | C+C+(D+A)+CARRYIN
      | C+C+(D+ACIN)
      | C+C+(D+ACIN)*(D+ACIN)
      | C+C+(D+ACIN)*(D+ACIN)+CARRYIN
      | C+C+(D+ACIN)*B
      | C+C+(D+ACIN)*B+CARRYIN
      | C+C+(D+ACIN)*BCIN
      | C+C+(D+ACIN)*BCIN+CARRYIN
      | C+C+(D+ACIN)+CARRYIN
      | C+C+(D-A)
      | C+C+(D-A)*(D-A)
      | C+C+(D-A)*(D-A)+CARRYIN
      | C+C+(D-A)*B
      | C+C+(D-A)*B+CARRYIN
      | C+C+(D-A)*BCIN
      | C+C+(D-A)*BCIN+CARRYIN
      | C+C+(D-A)+CARRYIN
      | C+C+(D-ACIN)
      | C+C+(D-ACIN)*(D-ACIN)
      | C+C+(D-ACIN)*(D-ACIN)+CARRYIN
      | C+C+(D-ACIN)*B
      | C+C+(D-ACIN)*B+CARRYIN
      | C+C+(D-ACIN)*BCIN
      | C+C+(D-ACIN)*BCIN+CARRYIN
      | C+C+(D-ACIN)+CARRYIN
      | C+C+A
      | C+C+A*A
      | C+C+A*A+CARRYIN
      | C+C+A*B
      | C+C+A*B+CARRYIN
      | C+C+A*BCIN
      | C+C+A*BCIN+CARRYIN
      | C+C+A+CARRYIN
      | C+C+ACIN
      | C+C+ACIN*ACIN
      | C+C+ACIN*ACIN+CARRYIN
      | C+C+ACIN*B
      | C+C+ACIN*B+CARRYIN
      | C+C+ACIN*BCIN
      | C+C+ACIN*BCIN+CARRYIN
      | C+C+ACIN+CARRYIN
      | C+C+B*(A+D)
      | C+C+B*(A+D)+CARRYIN
      | C+C+B*(ACIN+D)
      | C+C+B*(ACIN+D)+CARRYIN
      | C+C+B*(D+A)
      | C+C+B*(D+A)+CARRYIN
      | C+C+B*(D+ACIN)
      | C+C+B*(D+ACIN)+CARRYIN
      | C+C+B*(D-A)
      | C+C+B*(D-A)+CARRYIN
      | C+C+B*(D-ACIN)
      | C+C+B*(D-ACIN)+CARRYIN
      | C+C+B*A
      | C+C+B*A+CARRYIN
      | C+C+B*ACIN
      | C+C+B*ACIN+CARRYIN
      | C+C+B*D
      | C+C+B*D+CARRYIN
      | C+C+BCIN*(A+D)
      | C+C+BCIN*(A+D)+CARRYIN
      | C+C+BCIN*(ACIN+D)
      | C+C+BCIN*(ACIN+D)+CARRYIN
      | C+C+BCIN*(D+A)
      | C+C+BCIN*(D+A)+CARRYIN
      | C+C+BCIN*(D+ACIN)
      | C+C+BCIN*(D+ACIN)+CARRYIN
      | C+C+BCIN*(D-A)
      | C+C+BCIN*(D-A)+CARRYIN
      | C+C+BCIN*(D-ACIN)
      | C+C+BCIN*(D-ACIN)+CARRYIN
      | C+C+BCIN*A
      | C+C+BCIN*A+CARRYIN
      | C+C+BCIN*ACIN
      | C+C+BCIN*ACIN+CARRYIN
      | C+C+BCIN*D
      | C+C+BCIN*D+CARRYIN
      | C+C+C
      | C+C+C+CARRYIN
      | C+C+C+CONCAT
      | C+C+C+CONCAT+CARRYIN
      | C+C+C+P
      | C+C+C+P+CARRYIN
      | C+C+CARRYCASCIN
      | C+C+CARRYIN
      | C+C+CONCAT
      | C+C+CONCAT+C
      | C+C+CONCAT+C+CARRYIN
      | C+C+CONCAT+CARRYIN
      | C+C+CONCAT+P
      | C+C+CONCAT+P+CARRYIN
      | C+C+CONCAT+P>>17
      | C+C+CONCAT+P>>17+CARRYIN
      | C+C+CONCAT+PCIN
      | C+C+CONCAT+PCIN+CARRYIN
      | C+C+CONCAT+PCIN>>17
      | C+C+CONCAT+PCIN>>17+CARRYIN
      | C+C+CONCAT-C
      | C+C+CONCAT-P
      | C+C+CONCAT-P>>17
      | C+C+CONCAT-PCIN
      | C+C+CONCAT-PCIN>>17
      | C+C+D
      | C+C+D*B
      | C+C+D*B+CARRYIN
      | C+C+D*BCIN
      | C+C+D*BCIN+CARRYIN
      | C+C+D*D
      | C+C+D*D+CARRYIN
      | C+C+D+CARRYIN
      | C+C+P
      | C+C+P+C
      | C+C+P+C+CARRYIN
      | C+C+P+CARRYIN
      | C+C+P+CONCAT
      | C+C+P+CONCAT+CARRYIN
      | C+C+P+P
      | C+C+P+P+CARRYIN
      | C+C+P+P>>17
      | C+C+P+P>>17+CARRYIN
      | C+C+P+PCIN
      | C+C+P+PCIN+CARRYIN
      | C+C+P+PCIN>>17
      | C+C+P+PCIN>>17+CARRYIN
      | C+C+P-C
      | C+C+P-P
      | C+C+P-P>>17
      | C+C+P-PCIN
      | C+C+P-PCIN>>17
      | C+C+P>>17
      | C+C+P>>17+CARRYIN
      | C+C+P>>17+CONCAT
      | C+C+P>>17+CONCAT+CARRYIN
      | C+C+P>>17+P
      | C+C+P>>17+P+CARRYIN
      | C+C+PCIN
      | C+C+PCIN+CARRYIN
      | C+C+PCIN+CONCAT
      | C+C+PCIN+CONCAT+CARRYIN
      | C+C+PCIN+P
      | C+C+PCIN+P+CARRYIN
      | C+C+PCIN>>17
      | C+C+PCIN>>17+CARRYIN
      | C+C+PCIN>>17+CONCAT
      | C+C+PCIN>>17+CONCAT+CARRYIN
      | C+C+PCIN>>17+P
      | C+C+PCIN>>17+P+CARRYIN
      | C+C-C
      | C+C-P
      | C+C-P>>17
      | C+C-PCIN
      | C+C-PCIN>>17
      | C+CARRYCASCIN
      | C+CARRYIN
      | C+CONCAT
      | C+CONCAT+C
      | C+CONCAT+C+C
      | C+CONCAT+C+C+CARRYIN
      | C+CONCAT+C+CARRYIN
      | C+CONCAT+C+P
      | C+CONCAT+C+P+CARRYIN
      | C+CONCAT+C+P>>17
      | C+CONCAT+C+P>>17+CARRYIN
      | C+CONCAT+C+PCIN
      | C+CONCAT+C+PCIN+CARRYIN
      | C+CONCAT+C+PCIN>>17
      | C+CONCAT+C+PCIN>>17+CARRYIN
      | C+CONCAT+C-C
      | C+CONCAT+C-P
      | C+CONCAT+C-P>>17
      | C+CONCAT+C-PCIN
      | C+CONCAT+C-PCIN>>17
      | C+CONCAT+CARRYCASCIN
      | C+CONCAT+CARRYIN
      | C+CONCAT+P
      | C+CONCAT+P+C
      | C+CONCAT+P+C+CARRYIN
      | C+CONCAT+P+CARRYIN
      | C+CONCAT+P+P
      | C+CONCAT+P+P+CARRYIN
      | C+CONCAT+P+P>>17
      | C+CONCAT+P+P>>17+CARRYIN
      | C+CONCAT+P+PCIN
      | C+CONCAT+P+PCIN+CARRYIN
      | C+CONCAT+P+PCIN>>17
      | C+CONCAT+P+PCIN>>17+CARRYIN
      | C+CONCAT+P-C
      | C+CONCAT+P-P
      | C+CONCAT+P-P>>17
      | C+CONCAT+P-PCIN
      | C+CONCAT+P-PCIN>>17
      | C+CONCAT+P>>17
      | C+CONCAT+P>>17+C
      | C+CONCAT+P>>17+C+CARRYIN
      | C+CONCAT+P>>17+CARRYIN
      | C+CONCAT+P>>17+P
      | C+CONCAT+P>>17+P+CARRYIN
      | C+CONCAT+PCIN
      | C+CONCAT+PCIN+C
      | C+CONCAT+PCIN+C+CARRYIN
      | C+CONCAT+PCIN+CARRYIN
      | C+CONCAT+PCIN+P
      | C+CONCAT+PCIN+P+CARRYIN
      | C+CONCAT+PCIN>>17
      | C+CONCAT+PCIN>>17+C
      | C+CONCAT+PCIN>>17+C+CARRYIN
      | C+CONCAT+PCIN>>17+CARRYIN
      | C+CONCAT+PCIN>>17+P
      | C+CONCAT+PCIN>>17+P+CARRYIN
      | C+CONCAT-C
      | C+CONCAT-P
      | C+CONCAT-P>>17
      | C+CONCAT-PCIN
      | C+CONCAT-PCIN>>17
      | C+D
      | C+D*B
      | C+D*B+C
      | C+D*B+C+CARRYIN
      | C+D*B+CARRYIN
      | C+D*B+P
      | C+D*B+P+CARRYIN
      | C+D*B+P>>17
      | C+D*B+P>>17+CARRYIN
      | C+D*B+PCIN
      | C+D*B+PCIN+CARRYIN
      | C+D*B+PCIN>>17
      | C+D*B+PCIN>>17+CARRYIN
      | C+D*B-C
      | C+D*B-P
      | C+D*B-P>>17
      | C+D*B-PCIN
      | C+D*B-PCIN>>17
      | C+D*BCIN
      | C+D*BCIN+C
      | C+D*BCIN+C+CARRYIN
      | C+D*BCIN+CARRYIN
      | C+D*BCIN+P
      | C+D*BCIN+P+CARRYIN
      | C+D*BCIN+P>>17
      | C+D*BCIN+P>>17+CARRYIN
      | C+D*BCIN+PCIN
      | C+D*BCIN+PCIN+CARRYIN
      | C+D*BCIN+PCIN>>17
      | C+D*BCIN+PCIN>>17+CARRYIN
      | C+D*BCIN-C
      | C+D*BCIN-P
      | C+D*BCIN-P>>17
      | C+D*BCIN-PCIN
      | C+D*BCIN-PCIN>>17
      | C+D*D
      | C+D*D+C
      | C+D*D+C+CARRYIN
      | C+D*D+CARRYIN
      | C+D*D+P
      | C+D*D+P+CARRYIN
      | C+D*D+P>>17
      | C+D*D+P>>17+CARRYIN
      | C+D*D+PCIN
      | C+D*D+PCIN+CARRYIN
      | C+D*D+PCIN>>17
      | C+D*D+PCIN>>17+CARRYIN
      | C+D*D-C
      | C+D*D-P
      | C+D*D-P>>17
      | C+D*D-PCIN
      | C+D*D-PCIN>>17
      | C+D+C
      | C+D+C+CARRYIN
      | C+D+CARRYIN
      | C+D+P
      | C+D+P+CARRYIN
      | C+D+P>>17
      | C+D+P>>17+CARRYIN
      | C+D+PCIN
      | C+D+PCIN+CARRYIN
      | C+D+PCIN>>17
      | C+D+PCIN>>17+CARRYIN
      | C+D-C
      | C+D-P
      | C+D-P>>17
      | C+D-PCIN
      | C+D-PCIN>>17
      | C+P
      | C+P+(A+D)
      | C+P+(A+D)*(A+D)
      | C+P+(A+D)*(A+D)+CARRYIN
      | C+P+(A+D)*B
      | C+P+(A+D)*B+CARRYIN
      | C+P+(A+D)*BCIN
      | C+P+(A+D)*BCIN+CARRYIN
      | C+P+(A+D)+CARRYIN
      | C+P+(ACIN+D)
      | C+P+(ACIN+D)*(ACIN+D)
      | C+P+(ACIN+D)*(ACIN+D)+CARRYIN
      | C+P+(ACIN+D)*B
      | C+P+(ACIN+D)*B+CARRYIN
      | C+P+(ACIN+D)*BCIN
      | C+P+(ACIN+D)*BCIN+CARRYIN
      | C+P+(ACIN+D)+CARRYIN
      | C+P+(D+A)
      | C+P+(D+A)*(D+A)
      | C+P+(D+A)*(D+A)+CARRYIN
      | C+P+(D+A)*B
      | C+P+(D+A)*B+CARRYIN
      | C+P+(D+A)*BCIN
      | C+P+(D+A)*BCIN+CARRYIN
      | C+P+(D+A)+CARRYIN
      | C+P+(D+ACIN)
      | C+P+(D+ACIN)*(D+ACIN)
      | C+P+(D+ACIN)*(D+ACIN)+CARRYIN
      | C+P+(D+ACIN)*B
      | C+P+(D+ACIN)*B+CARRYIN
      | C+P+(D+ACIN)*BCIN
      | C+P+(D+ACIN)*BCIN+CARRYIN
      | C+P+(D+ACIN)+CARRYIN
      | C+P+(D-A)
      | C+P+(D-A)*(D-A)
      | C+P+(D-A)*(D-A)+CARRYIN
      | C+P+(D-A)*B
      | C+P+(D-A)*B+CARRYIN
      | C+P+(D-A)*BCIN
      | C+P+(D-A)*BCIN+CARRYIN
      | C+P+(D-A)+CARRYIN
      | C+P+(D-ACIN)
      | C+P+(D-ACIN)*(D-ACIN)
      | C+P+(D-ACIN)*(D-ACIN)+CARRYIN
      | C+P+(D-ACIN)*B
      | C+P+(D-ACIN)*B+CARRYIN
      | C+P+(D-ACIN)*BCIN
      | C+P+(D-ACIN)*BCIN+CARRYIN
      | C+P+(D-ACIN)+CARRYIN
      | C+P+A
      | C+P+A*A
      | C+P+A*A+CARRYIN
      | C+P+A*B
      | C+P+A*B+CARRYIN
      | C+P+A*BCIN
      | C+P+A*BCIN+CARRYIN
      | C+P+A+CARRYIN
      | C+P+ACIN
      | C+P+ACIN*ACIN
      | C+P+ACIN*ACIN+CARRYIN
      | C+P+ACIN*B
      | C+P+ACIN*B+CARRYIN
      | C+P+ACIN*BCIN
      | C+P+ACIN*BCIN+CARRYIN
      | C+P+ACIN+CARRYIN
      | C+P+B*(A+D)
      | C+P+B*(A+D)+CARRYIN
      | C+P+B*(ACIN+D)
      | C+P+B*(ACIN+D)+CARRYIN
      | C+P+B*(D+A)
      | C+P+B*(D+A)+CARRYIN
      | C+P+B*(D+ACIN)
      | C+P+B*(D+ACIN)+CARRYIN
      | C+P+B*(D-A)
      | C+P+B*(D-A)+CARRYIN
      | C+P+B*(D-ACIN)
      | C+P+B*(D-ACIN)+CARRYIN
      | C+P+B*A
      | C+P+B*A+CARRYIN
      | C+P+B*ACIN
      | C+P+B*ACIN+CARRYIN
      | C+P+B*D
      | C+P+B*D+CARRYIN
      | C+P+BCIN*(A+D)
      | C+P+BCIN*(A+D)+CARRYIN
      | C+P+BCIN*(ACIN+D)
      | C+P+BCIN*(ACIN+D)+CARRYIN
      | C+P+BCIN*(D+A)
      | C+P+BCIN*(D+A)+CARRYIN
      | C+P+BCIN*(D+ACIN)
      | C+P+BCIN*(D+ACIN)+CARRYIN
      | C+P+BCIN*(D-A)
      | C+P+BCIN*(D-A)+CARRYIN
      | C+P+BCIN*(D-ACIN)
      | C+P+BCIN*(D-ACIN)+CARRYIN
      | C+P+BCIN*A
      | C+P+BCIN*A+CARRYIN
      | C+P+BCIN*ACIN
      | C+P+BCIN*ACIN+CARRYIN
      | C+P+BCIN*D
      | C+P+BCIN*D+CARRYIN
      | C+P+C
      | C+P+C+C
      | C+P+C+C+CARRYIN
      | C+P+C+CARRYIN
      | C+P+C+CONCAT
      | C+P+C+CONCAT+CARRYIN
      | C+P+C+P
      | C+P+C+P+CARRYIN
      | C+P+C+P>>17
      | C+P+C+P>>17+CARRYIN
      | C+P+C+PCIN
      | C+P+C+PCIN+CARRYIN
      | C+P+C+PCIN>>17
      | C+P+C+PCIN>>17+CARRYIN
      | C+P+C-C
      | C+P+C-P
      | C+P+C-P>>17
      | C+P+C-PCIN
      | C+P+C-PCIN>>17
      | C+P+CARRYCASCIN
      | C+P+CARRYIN
      | C+P+CONCAT
      | C+P+CONCAT+C
      | C+P+CONCAT+C+CARRYIN
      | C+P+CONCAT+CARRYIN
      | C+P+CONCAT+P
      | C+P+CONCAT+P+CARRYIN
      | C+P+CONCAT+P>>17
      | C+P+CONCAT+P>>17+CARRYIN
      | C+P+CONCAT+PCIN
      | C+P+CONCAT+PCIN+CARRYIN
      | C+P+CONCAT+PCIN>>17
      | C+P+CONCAT+PCIN>>17+CARRYIN
      | C+P+CONCAT-C
      | C+P+CONCAT-P
      | C+P+CONCAT-P>>17
      | C+P+CONCAT-PCIN
      | C+P+CONCAT-PCIN>>17
      | C+P+D
      | C+P+D*B
      | C+P+D*B+CARRYIN
      | C+P+D*BCIN
      | C+P+D*BCIN+CARRYIN
      | C+P+D*D
      | C+P+D*D+CARRYIN
      | C+P+D+CARRYIN
      | C+P+P
      | C+P+P+C
      | C+P+P+C+CARRYIN
      | C+P+P+CARRYIN
      | C+P+P+CONCAT
      | C+P+P+CONCAT+CARRYIN
      | C+P+P+P
      | C+P+P+P+CARRYIN
      | C+P+P+P>>17
      | C+P+P+P>>17+CARRYIN
      | C+P+P+PCIN
      | C+P+P+PCIN+CARRYIN
      | C+P+P+PCIN>>17
      | C+P+P+PCIN>>17+CARRYIN
      | C+P+P-C
      | C+P+P-P
      | C+P+P-P>>17
      | C+P+P-PCIN
      | C+P+P-PCIN>>17
      | C+P+P>>17
      | C+P+P>>17+C
      | C+P+P>>17+C+CARRYIN
      | C+P+P>>17+CARRYIN
      | C+P+P>>17+CONCAT
      | C+P+P>>17+CONCAT+CARRYIN
      | C+P+P>>17+P
      | C+P+P>>17+P+CARRYIN
      | C+P+PCIN
      | C+P+PCIN+C
      | C+P+PCIN+C+CARRYIN
      | C+P+PCIN+CARRYIN
      | C+P+PCIN+CONCAT
      | C+P+PCIN+CONCAT+CARRYIN
      | C+P+PCIN+P
      | C+P+PCIN+P+CARRYIN
      | C+P+PCIN>>17
      | C+P+PCIN>>17+C
      | C+P+PCIN>>17+C+CARRYIN
      | C+P+PCIN>>17+CARRYIN
      | C+P+PCIN>>17+CONCAT
      | C+P+PCIN>>17+CONCAT+CARRYIN
      | C+P+PCIN>>17+P
      | C+P+PCIN>>17+P+CARRYIN
      | C+P-C
      | C+P-P
      | C+P-P>>17
      | C+P-PCIN
      | C+P-PCIN>>17
      | C+P>>17
      | C+P>>17+(A+D)
      | C+P>>17+(A+D)*(A+D)
      | C+P>>17+(A+D)*(A+D)+CARRYIN
      | C+P>>17+(A+D)*B
      | C+P>>17+(A+D)*B+CARRYIN
      | C+P>>17+(A+D)*BCIN
      | C+P>>17+(A+D)*BCIN+CARRYIN
      | C+P>>17+(A+D)+CARRYIN
      | C+P>>17+(ACIN+D)
      | C+P>>17+(ACIN+D)*(ACIN+D)
      | C+P>>17+(ACIN+D)*(ACIN+D)+CARRYIN
      | C+P>>17+(ACIN+D)*B
      | C+P>>17+(ACIN+D)*B+CARRYIN
      | C+P>>17+(ACIN+D)*BCIN
      | C+P>>17+(ACIN+D)*BCIN+CARRYIN
      | C+P>>17+(ACIN+D)+CARRYIN
      | C+P>>17+(D+A)
      | C+P>>17+(D+A)*(D+A)
      | C+P>>17+(D+A)*(D+A)+CARRYIN
      | C+P>>17+(D+A)*B
      | C+P>>17+(D+A)*B+CARRYIN
      | C+P>>17+(D+A)*BCIN
      | C+P>>17+(D+A)*BCIN+CARRYIN
      | C+P>>17+(D+A)+CARRYIN
      | C+P>>17+(D+ACIN)
      | C+P>>17+(D+ACIN)*(D+ACIN)
      | C+P>>17+(D+ACIN)*(D+ACIN)+CARRYIN
      | C+P>>17+(D+ACIN)*B
      | C+P>>17+(D+ACIN)*B+CARRYIN
      | C+P>>17+(D+ACIN)*BCIN
      | C+P>>17+(D+ACIN)*BCIN+CARRYIN
      | C+P>>17+(D+ACIN)+CARRYIN
      | C+P>>17+(D-A)
      | C+P>>17+(D-A)*(D-A)
      | C+P>>17+(D-A)*(D-A)+CARRYIN
      | C+P>>17+(D-A)*B
      | C+P>>17+(D-A)*B+CARRYIN
      | C+P>>17+(D-A)*BCIN
      | C+P>>17+(D-A)*BCIN+CARRYIN
      | C+P>>17+(D-A)+CARRYIN
      | C+P>>17+(D-ACIN)
      | C+P>>17+(D-ACIN)*(D-ACIN)
      | C+P>>17+(D-ACIN)*(D-ACIN)+CARRYIN